simulink model "mbf_3beams"  (R2013b)
settings: Solver=VariableStepDiscrete  StartTime=0.0  StopTime=1000  RelTol=1e-3
undefined symbols (not defined in the file): pfb_coeff_gen_calc
libraries: xbsIndex_r4 x21411, casper_library_misc x306, casper_library_delays x93, casper_library_multipliers x64, casper_library_pfbs x16, simulink x16, xbsReplacements_r4 x8, xps_library x1
(part 2 of 252)

== system mbf_3beams/acc_control ==
  sync : Inport
  acc_len : Inport  Port=2
  rst : Inport  Port=3
  new_acc : Inport  Port=4
  valid : Inport  Port=5
  Counter : xbsIndex_r4/Counter  cnt_type=Count Limited  cnt_to=255  operation=Up  start_count=0  cnt_by_val=1  arith_type=Unsigned  n_bits=8  bin_pt=0  load_pin=off  rst=on  en=on  explicit_period=on  period=1  dbl_ovrd=off  use_behavioral_HDL=off  implementation=Fabric  block_type=counter
  Counter1 : xbsIndex_r4/Counter  cnt_type=Free Running  cnt_to=Inf  operation=Up  start_count=0  cnt_by_val=1  arith_type=Unsigned  n_bits=32  bin_pt=0  load_pin=off  rst=on  en=on  explicit_period=on  period=1  dbl_ovrd=off  use_behavioral_HDL=off  implementation=Fabric  block_type=counter
  acc_cntrl : Subsystem  mask{chan_bits=8}
      mask prompts: chan_bits "Number of Channels (2^?)"
  acc_control : Subsystem
  acc_count : Subsystem  Tag=xps:sw_reg  AncestorBlock=xps_library/software_register  mask(swreg){io_dir=To Processor, io_delay=0, sample_period=1, names=reg, bitwidths=32, bin_pts=0, arith_types=0, sim_port=off, show_format=off}
      mask prompts: io_dir "I/O direction"; io_delay "I/O delay"; sample_period "Sample period"; names "Bitfield names [msb...lsb]"; bitwidths "Bitfield widths"; bin_pts "Bitfield binary pts"; arith_types "Bitfield types, ufix=0, fix=1, bool=2"; sim_port "Provide sim input/output?"; show_format "Print format string?"
      mask help: A 32-bit software-accessible register. Can be divided into bitfields of varying widths and types using the fields in the block mask.
      mask init:
      | try
      |     swreg_init(gcb)
      | catch ex
      |     disp(sprintf('%s: %s', gcb, ex.message))
      |     errordlg(ex.message)
      | end
      settings: AttributesFormatString=1output
      mask TypeOptions io_dir: To Processor | From Processor
      mask Callback io_dir:
      | mask_enables = get_param(gcb, 'MaskEnables');
      | mask_names = get_param(gcb, 'MaskNames');
      | if strcmp(get_param(gcb, 'io_dir'), 'To Processor'),
      |     mask_enables{ismember(mask_names, 'sample_period')} = 'off';
      | else
      |     mask_enables{ismember(mask_names, 'sample_period')} = 'on';
      | end
      | set_param(gcb, 'MaskEnables', mask_enables);
  edge_detect : Subsystem  AncestorBlock=casper_library_misc/edge_detect  mask(edge_detect){edge=Falling, polarity=Active High, x_in=[0 0.08 0.08 0.16 0.16 0.24 0.24 0.32 0.32 0.4], y_in=[-1 -1  1  1  1  1  1  1  1  1], x_out=[0.6 0.68 0.68 0.76 0.76 0.84 0.84 0.92 0.92 1], y_out=[-1 -1  1  1 -1 -1 -1 -1 -1 -1]}
      mask prompts: edge "Edge Type"; polarity "Output Polarity"; x_in "Input X Positions"; y_in "Input Y Positions"; x_out "Output X Positions"; y_out "Output Y Positions"
      mask help: Output is active for one-cycle after each edge of the specified type (i.e. rising, falling or both).
      mask init:
      | edge_detect_init(gcb,...
      |     'edge',edge,...
      |     'polarity',polarity,...
      |     'x_in',x_in,...
      |     'x_out',x_out,...
      |     'y_in',y_in,...
      |     'y_out',y_out);
      | 
      | x_in_num=str2num(getfield(getfield(get_param(gcb,'UserData'),'parameters'),'x_in'));
      | x_out_num=str2num(getfield(getfield(get_param(gcb,'UserData'),'parameters'),'x_out'));
      | y_in_num=str2num(getfield(getfield(get_param(gcb,'UserData'),'parameters'),'y_in'));
      | y_out_num=str2num(getfield(getfield(get_param(gcb,'UserData'),'parameters'),'y_out'));
      settings: AttributesFormatString=|
      | falling edges
      | active high
      mask Help: Output is active for one-cycle after each edge of the specified type (i.e. rising, falling, or both).  Latency is zero.
      mask TypeOptions edge: Rising | Falling | Both
      mask TypeOptions polarity: Active High | Active Low
      mask Display:
      | plot(x_in_num,0.5+y_in_num*0.2);
      | plot(x_out_num,0.5+y_out_num*0.2);
  edge_detect1 : Subsystem  AncestorBlock=casper_library_misc/edge_detect  mask(edge_detect){edge=Rising, polarity=Active High, x_in=[0 0.08 0.08 0.16 0.16 0.24 0.24 0.32 0.32 0.4], y_in=[-1 -1  1  1  1  1  1  1  1  1], x_out=[0.6 0.68 0.68 0.76 0.76 0.84 0.84 0.92 0.92 1], y_out=[-1 -1  1  1 -1 -1 -1 -1 -1 -1]}
      mask prompts: edge "Edge Type"; polarity "Output Polarity"; x_in "Input X Positions"; y_in "Input Y Positions"; x_out "Output X Positions"; y_out "Output Y Positions"
      mask help: Output is active for one-cycle after each edge of the specified type (i.e. rising, falling or both).
      mask init: same as mbf_3beams/acc_control/edge_detect
      settings: AttributesFormatString=|
      | rising edges
      | active high
      mask Help: Output is active for one-cycle after each edge of the specified type (i.e. rising, falling, or both).  Latency is zero.
      mask TypeOptions edge: Rising | Falling | Both
      mask TypeOptions polarity: Active High | Active Low
      mask Display:
      | plot(x_in_num,0.5+y_in_num*0.2);
      | plot(x_out_num,0.5+y_out_num*0.2);
  edge_detect3 : Subsystem  AncestorBlock=casper_library_misc/edge_detect  mask(edge_detect){edge=Rising, polarity=Active High, x_in=[0 0.08 0.08 0.16 0.16 0.24 0.24 0.32 0.32 0.4], y_in=[-1 -1  1  1  1  1  1  1  1  1], x_out=[0.6 0.68 0.68 0.76 0.76 0.84 0.84 0.92 0.92 1], y_out=[-1 -1  1  1 -1 -1 -1 -1 -1 -1]}
      mask prompts: edge "Edge Type"; polarity "Output Polarity"; x_in "Input X Positions"; y_in "Input Y Positions"; x_out "Output X Positions"; y_out "Output Y Positions"
      mask help: Output is active for one-cycle after each edge of the specified type (i.e. rising, falling or both).
      mask init: same as mbf_3beams/acc_control/edge_detect
      settings: AttributesFormatString=|
      | rising edges
      | active high
      mask Help: Output is active for one-cycle after each edge of the specified type (i.e. rising, falling, or both).  Latency is zero.
      mask TypeOptions edge: Rising | Falling | Both
      mask TypeOptions polarity: Active High | Active Low
      mask Display:
      | plot(x_in_num,0.5+y_in_num*0.2);
      | plot(x_out_num,0.5+y_out_num*0.2);
  pipeline214 : Subsystem  AncestorBlock=casper_library_delays/pipeline  mask(pipeline){latency=1}
      mask help:
      | An explicitly laid-out delay line to avoid mapping to SRL16s
      | for use in pipelining to help achieve timing closure.
      mask init:
      | pipeline_init(gcb,'latency',latency);
      settings: AttributesFormatString=Latency = %<latency>
      mask Display:
      | port_label('input', 1, 'd');
      | port_label('output', 1, 'q');
      | disp(['Z^-', num2str(latency)]);
  pipeline50 : Subsystem  AncestorBlock=casper_library_delays/pipeline  mask(pipeline){latency=1}
      mask help:
      | An explicitly laid-out delay line to avoid mapping to SRL16s
      | for use in pipelining to help achieve timing closure.
      mask init:
      | pipeline_init(gcb,'latency',latency);
      settings: AttributesFormatString=Latency = %<latency>
      mask Display:
      | port_label('input', 1, 'd');
      | port_label('output', 1, 'q');
      | disp(['Z^-', num2str(latency)]);
  rst (dup) : InportShadow  Port=3
  ctrl_new_acc : Outport
  ctrl_addr : Outport  Port=2
  ctrl_we : Outport  Port=3
  wires:
  Counter1/1 -> acc_count/1(out_reg)
  sync/1 -> acc_cntrl/2(sync)
  acc_len/1 -> acc_cntrl/1(acc_len)
  rst/1 -> acc_cntrl/3(rst)
  acc_cntrl/1(new_acc) -> ctrl_new_acc/1
  edge_detect/1(out) -> Counter1/2
  Counter/1 -> ctrl_addr/1
  edge_detect1/1(out) -> Counter/1
  pipeline50/1(q) -> Counter/2
  pipeline214/1(q) -> ctrl_we/1
  acc_control/1(cal_we) -> edge_detect/1(in), edge_detect1/1(in), pipeline50/1(d), pipeline214/1(d)
  new_acc/1 -> edge_detect3/1(in)
  edge_detect3/1(out) -> acc_control/1(new_acc)
  valid/1 -> acc_control/2(valid)
  rst (dup)/1 -> Counter1/1

== system mbf_3beams/acc_control_bf ==
  sync : Inport
  acc_len : Inport  Port=2
  rst : Inport  Port=3
  new_acc : Inport  Port=4
  valid : Inport  Port=5
  Counter : xbsIndex_r4/Counter  cnt_type=Count Limited  cnt_to=255  operation=Up  start_count=0  cnt_by_val=1  arith_type=Unsigned  n_bits=8  bin_pt=0  load_pin=off  rst=on  en=on  explicit_period=on  period=1  dbl_ovrd=off  use_behavioral_HDL=off  implementation=Fabric  block_type=counter
  Counter1 : xbsIndex_r4/Counter  cnt_type=Free Running  cnt_to=Inf  operation=Up  start_count=0  cnt_by_val=1  arith_type=Unsigned  n_bits=32  bin_pt=0  load_pin=off  rst=on  en=on  explicit_period=on  period=1  dbl_ovrd=off  use_behavioral_HDL=off  implementation=Fabric  block_type=counter
  acc_cntrl : Subsystem  mask{chan_bits=8}
      mask prompts: chan_bits "Number of Channels (2^?)"
  acc_control : Subsystem
  acc_count : Subsystem  Tag=xps:sw_reg  AncestorBlock=xps_library/software_register  mask(swreg){io_dir=To Processor, io_delay=0, sample_period=1, names=reg, bitwidths=32, bin_pts=0, arith_types=0, sim_port=off, show_format=off}
      mask prompts: io_dir "I/O direction"; io_delay "I/O delay"; sample_period "Sample period"; names "Bitfield names [msb...lsb]"; bitwidths "Bitfield widths"; bin_pts "Bitfield binary pts"; arith_types "Bitfield types, ufix=0, fix=1, bool=2"; sim_port "Provide sim input/output?"; show_format "Print format string?"
      mask help: A 32-bit software-accessible register. Can be divided into bitfields of varying widths and types using the fields in the block mask.
      mask init:
      | try
      |     swreg_init(gcb)
      | catch ex
      |     disp(sprintf('%s: %s', gcb, ex.message))
      |     errordlg(ex.message)
      | end
      settings: AttributesFormatString=1output
      mask TypeOptions io_dir: To Processor | From Processor
      mask Callback io_dir:
      | mask_enables = get_param(gcb, 'MaskEnables');
      | mask_names = get_param(gcb, 'MaskNames');
      | if strcmp(get_param(gcb, 'io_dir'), 'To Processor'),
      |     mask_enables{ismember(mask_names, 'sample_period')} = 'off';
      | else
      |     mask_enables{ismember(mask_names, 'sample_period')} = 'on';
      | end
      | set_param(gcb, 'MaskEnables', mask_enables);
  edge_detect : Subsystem  AncestorBlock=casper_library_misc/edge_detect  mask(edge_detect){edge=Falling, polarity=Active High, x_in=[0 0.08 0.08 0.16 0.16 0.24 0.24 0.32 0.32 0.4], y_in=[-1 -1  1  1  1  1  1  1  1  1], x_out=[0.6 0.68 0.68 0.76 0.76 0.84 0.84 0.92 0.92 1], y_out=[-1 -1  1  1 -1 -1 -1 -1 -1 -1]}
      mask prompts: edge "Edge Type"; polarity "Output Polarity"; x_in "Input X Positions"; y_in "Input Y Positions"; x_out "Output X Positions"; y_out "Output Y Positions"
      mask help: Output is active for one-cycle after each edge of the specified type (i.e. rising, falling or both).
      mask init: same as mbf_3beams/acc_control/edge_detect
      settings: AttributesFormatString=|
      | falling edges
      | active high
      mask Help: Output is active for one-cycle after each edge of the specified type (i.e. rising, falling, or both).  Latency is zero.
      mask TypeOptions edge: Rising | Falling | Both
      mask TypeOptions polarity: Active High | Active Low
      mask Display:
      | plot(x_in_num,0.5+y_in_num*0.2);
      | plot(x_out_num,0.5+y_out_num*0.2);
  edge_detect1 : Subsystem  AncestorBlock=casper_library_misc/edge_detect  mask(edge_detect){edge=Rising, polarity=Active High, x_in=[0 0.08 0.08 0.16 0.16 0.24 0.24 0.32 0.32 0.4], y_in=[-1 -1  1  1  1  1  1  1  1  1], x_out=[0.6 0.68 0.68 0.76 0.76 0.84 0.84 0.92 0.92 1], y_out=[-1 -1  1  1 -1 -1 -1 -1 -1 -1]}
      mask prompts: edge "Edge Type"; polarity "Output Polarity"; x_in "Input X Positions"; y_in "Input Y Positions"; x_out "Output X Positions"; y_out "Output Y Positions"
      mask help: Output is active for one-cycle after each edge of the specified type (i.e. rising, falling or both).
      mask init: same as mbf_3beams/acc_control/edge_detect
      settings: AttributesFormatString=|
      | rising edges
      | active high
      mask Help: Output is active for one-cycle after each edge of the specified type (i.e. rising, falling, or both).  Latency is zero.
      mask TypeOptions edge: Rising | Falling | Both
      mask TypeOptions polarity: Active High | Active Low
      mask Display:
      | plot(x_in_num,0.5+y_in_num*0.2);
      | plot(x_out_num,0.5+y_out_num*0.2);
  edge_detect3 : Subsystem  AncestorBlock=casper_library_misc/edge_detect  mask(edge_detect){edge=Rising, polarity=Active High, x_in=[0 0.08 0.08 0.16 0.16 0.24 0.24 0.32 0.32 0.4], y_in=[-1 -1  1  1  1  1  1  1  1  1], x_out=[0.6 0.68 0.68 0.76 0.76 0.84 0.84 0.92 0.92 1], y_out=[-1 -1  1  1 -1 -1 -1 -1 -1 -1]}
      mask prompts: edge "Edge Type"; polarity "Output Polarity"; x_in "Input X Positions"; y_in "Input Y Positions"; x_out "Output X Positions"; y_out "Output Y Positions"
      mask help: Output is active for one-cycle after each edge of the specified type (i.e. rising, falling or both).
      mask init: same as mbf_3beams/acc_control/edge_detect
      settings: AttributesFormatString=|
      | rising edges
      | active high
      mask Help: Output is active for one-cycle after each edge of the specified type (i.e. rising, falling, or both).  Latency is zero.
      mask TypeOptions edge: Rising | Falling | Both
      mask TypeOptions polarity: Active High | Active Low
      mask Display:
      | plot(x_in_num,0.5+y_in_num*0.2);
      | plot(x_out_num,0.5+y_out_num*0.2);
  pipeline214 : Subsystem  AncestorBlock=casper_library_delays/pipeline  mask(pipeline){latency=1}
      mask help:
      | An explicitly laid-out delay line to avoid mapping to SRL16s
      | for use in pipelining to help achieve timing closure.
      mask init:
      | pipeline_init(gcb,'latency',latency);
      settings: AttributesFormatString=Latency = %<latency>
      mask Display:
      | port_label('input', 1, 'd');
      | port_label('output', 1, 'q');
      | disp(['Z^-', num2str(latency)]);
  pipeline50 : Subsystem  AncestorBlock=casper_library_delays/pipeline  mask(pipeline){latency=1}
      mask help:
      | An explicitly laid-out delay line to avoid mapping to SRL16s
      | for use in pipelining to help achieve timing closure.
      mask init:
      | pipeline_init(gcb,'latency',latency);
      settings: AttributesFormatString=Latency = %<latency>
      mask Display:
      | port_label('input', 1, 'd');
      | port_label('output', 1, 'q');
      | disp(['Z^-', num2str(latency)]);
  rst (dup) : InportShadow  Port=3
  ctrl_new_acc : Outport
  ctrl_addr : Outport  Port=2
  ctrl_we : Outport  Port=3
  wires:
  Counter1/1 -> acc_count/1(out_reg)
  sync/1 -> acc_cntrl/2(sync)
  acc_len/1 -> acc_cntrl/1(acc_len)
  rst/1 -> acc_cntrl/3(rst)
  acc_cntrl/1(new_acc) -> ctrl_new_acc/1
  edge_detect/1(out) -> Counter1/2
  Counter/1 -> ctrl_addr/1
  edge_detect1/1(out) -> Counter/1
  pipeline50/1(q) -> Counter/2
  pipeline214/1(q) -> ctrl_we/1
  acc_control/1(cal_we) -> edge_detect/1(in), edge_detect1/1(in), pipeline50/1(d), pipeline214/1(d)
  new_acc/1 -> edge_detect3/1(in)
  edge_detect3/1(out) -> acc_control/1(new_acc)
  valid/1 -> acc_control/2(valid)
  rst (dup)/1 -> Counter1/1

== system mbf_3beams/adc16x250-8 ==
  a1_sim : Inport
  a2_sim : Inport  Port=2
  a3_sim : Inport  Port=3
  a4_sim : Inport  Port=4
  b1_sim : Inport  Port=5
  b2_sim : Inport  Port=6
  b3_sim : Inport  Port=7
  b4_sim : Inport  Port=8
  c1_sim : Inport  Port=9
  c2_sim : Inport  Port=10
  c3_sim : Inport  Port=11
  c4_sim : Inport  Port=12
  d1_sim : Inport  Port=13
  d2_sim : Inport  Port=14
  d3_sim : Inport  Port=15
  d4_sim : Inport  Port=16
  mbf_3beams_adc16x250_8_a{1..4} (4 alike) : xbsIndex_r4/Gateway In  gui_display_data_type=Fixed-point  arith_type="Signed  (2's comp)"  n_bits=8  bin_pt=7  preci_type=Single  exp_width=8  frac_width=24  quantization="Round  (unbiased: +/- Inf)"  overflow=Saturate  period=1  dbl_ovrd=off  timing_constraint=None  locs_specified=off  LOCs={}  UseAsADC=off  ADCChannel='1'  inherit_from_input=off  hdl_port=on  block_type=gatewayin
  mbf_3beams_adc16x250_8_b{1..4} (4 alike) : xbsIndex_r4/Gateway In  gui_display_data_type=Fixed-point  arith_type="Signed  (2's comp)"  n_bits=8  bin_pt=7  preci_type=Single  exp_width=8  frac_width=24  quantization="Round  (unbiased: +/- Inf)"  overflow=Saturate  period=1  dbl_ovrd=off  timing_constraint=None  locs_specified=off  LOCs={}  UseAsADC=off  ADCChannel='1'  inherit_from_input=off  hdl_port=on  block_type=gatewayin
  mbf_3beams_adc16x250_8_c{1..4} (4 alike) : xbsIndex_r4/Gateway In  gui_display_data_type=Fixed-point  arith_type="Signed  (2's comp)"  n_bits=8  bin_pt=7  preci_type=Single  exp_width=8  frac_width=24  quantization="Round  (unbiased: +/- Inf)"  overflow=Saturate  period=1  dbl_ovrd=off  timing_constraint=None  locs_specified=off  LOCs={}  UseAsADC=off  ADCChannel='1'  inherit_from_input=off  hdl_port=on  block_type=gatewayin
  mbf_3beams_adc16x250_8_d{1..4} (4 alike) : xbsIndex_r4/Gateway In  gui_display_data_type=Fixed-point  arith_type="Signed  (2's comp)"  n_bits=8  bin_pt=7  preci_type=Single  exp_width=8  frac_width=24  quantization="Round  (unbiased: +/- Inf)"  overflow=Saturate  period=1  dbl_ovrd=off  timing_constraint=None  locs_specified=off  LOCs={}  UseAsADC=off  ADCChannel='1'  inherit_from_input=off  hdl_port=on  block_type=gatewayin
  a1 : Outport
  a2 : Outport  Port=2
  a3 : Outport  Port=3
  a4 : Outport  Port=4
  b1 : Outport  Port=5
  b2 : Outport  Port=6
  b3 : Outport  Port=7
  b4 : Outport  Port=8
  c1 : Outport  Port=9
  c2 : Outport  Port=10
  c3 : Outport  Port=11
  c4 : Outport  Port=12
  d1 : Outport  Port=13
  d2 : Outport  Port=14
  d3 : Outport  Port=15
  d4 : Outport  Port=16
  wires:
  a1_sim/1 -> mbf_3beams_adc16x250_8_a1/1
  mbf_3beams_adc16x250_8_a1/1 -> a1/1  "a1"
  a2_sim/1 -> mbf_3beams_adc16x250_8_a2/1
  mbf_3beams_adc16x250_8_a2/1 -> a2/1  "a2"
  a3_sim/1 -> mbf_3beams_adc16x250_8_a3/1
  mbf_3beams_adc16x250_8_a3/1 -> a3/1  "a3"
  a4_sim/1 -> mbf_3beams_adc16x250_8_a4/1
  mbf_3beams_adc16x250_8_a4/1 -> a4/1  "a4"
  b1_sim/1 -> mbf_3beams_adc16x250_8_b1/1
  mbf_3beams_adc16x250_8_b1/1 -> b1/1  "b1"
  b2_sim/1 -> mbf_3beams_adc16x250_8_b2/1
  mbf_3beams_adc16x250_8_b2/1 -> b2/1  "b2"
  b3_sim/1 -> mbf_3beams_adc16x250_8_b3/1
  mbf_3beams_adc16x250_8_b3/1 -> b3/1  "b3"
  b4_sim/1 -> mbf_3beams_adc16x250_8_b4/1
  mbf_3beams_adc16x250_8_b4/1 -> b4/1  "b4"
  c1_sim/1 -> mbf_3beams_adc16x250_8_c1/1
  mbf_3beams_adc16x250_8_c1/1 -> c1/1  "c1"
  c2_sim/1 -> mbf_3beams_adc16x250_8_c2/1
  mbf_3beams_adc16x250_8_c2/1 -> c2/1  "c2"
  c3_sim/1 -> mbf_3beams_adc16x250_8_c3/1
  mbf_3beams_adc16x250_8_c3/1 -> c3/1  "c3"
  c4_sim/1 -> mbf_3beams_adc16x250_8_c4/1
  mbf_3beams_adc16x250_8_c4/1 -> c4/1  "c4"
  d1_sim/1 -> mbf_3beams_adc16x250_8_d1/1
  mbf_3beams_adc16x250_8_d1/1 -> d1/1  "d1"
  d2_sim/1 -> mbf_3beams_adc16x250_8_d2/1
  mbf_3beams_adc16x250_8_d2/1 -> d2/1  "d2"
  d3_sim/1 -> mbf_3beams_adc16x250_8_d3/1
  mbf_3beams_adc16x250_8_d3/1 -> d3/1  "d3"
  d4_sim/1 -> mbf_3beams_adc16x250_8_d4/1
  mbf_3beams_adc16x250_8_d4/1 -> d4/1  "d4"

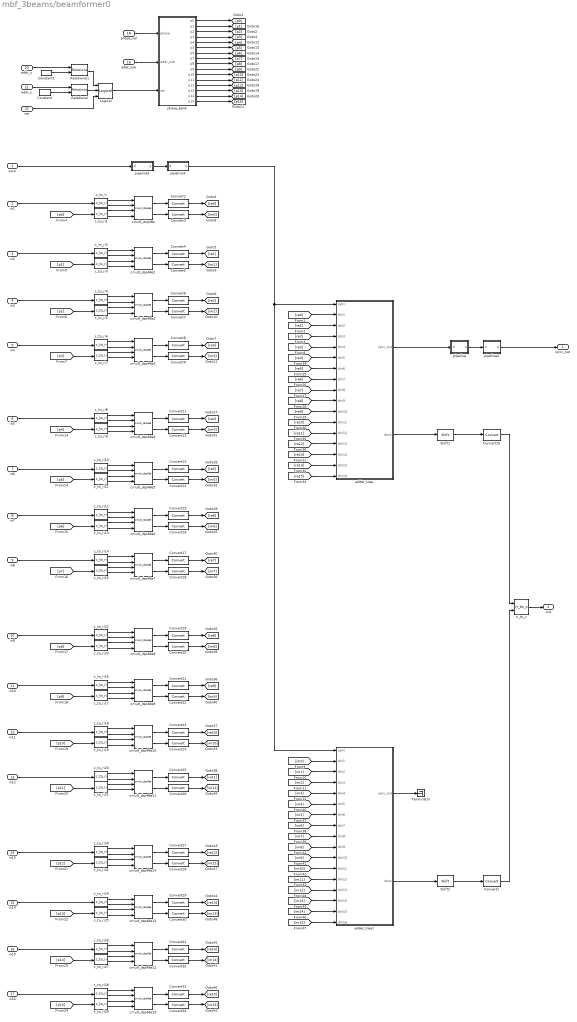
== system mbf_3beams/beamformer0 ==
  sync : Inport
  in1 : Inport  Port=2
  in2 : Inport  Port=3
  in3 : Inport  Port=4
  in4 : Inport  Port=5
  in5 : Inport  Port=6
  in6 : Inport  Port=7
  in7 : Inport  Port=8
  in8 : Inport  Port=9
  in9 : Inport  Port=10
  in10 : Inport  Port=11
  in11 : Inport  Port=12
  in12 : Inport  Port=13
  in13 : Inport  Port=14
  in14 : Inport  Port=15
  in15 : Inport  Port=16
  in16 : Inport  Port=17
  phase_val : Inport  Port=18
  addr_sub : Inport  Port=19
  addr_x : Inport  Port=20
  addr_y : Inport  Port=21
  we : Inport  Port=22
  Constant : xbsIndex_r4/Constant  const=addr_y  gui_display_data_type=Fixed-point  arith_type=Unsigned  n_bits=32  bin_pt=0  preci_type=Single  exp_width=8  frac_width=24  explicit_period=on  period=1  equ=P=C  opselect=C  inp2=PCIN>>17  opr=+  inp1=P  carry=CIN  dbl_ovrd=off  block_type=constant
  Constant1 : xbsIndex_r4/Constant  const=addr_x  gui_display_data_type=Fixed-point  arith_type=Unsigned  n_bits=32  bin_pt=0  preci_type=Single  exp_width=8  frac_width=24  explicit_period=on  period=1  equ=P=C  opselect=C  inp2=PCIN>>17  opr=+  inp1=P  carry=CIN  dbl_ovrd=off  block_type=constant
  Convert{1..34} (34 alike) : xbsIndex_r4/Convert  gui_display_data_type=Fixed-point  arith_type="Signed  (2's comp)"  n_bits=18  bin_pt=17  float_type=Single  exp_bits=8  fraction_bits=24  quantization=Truncate  overflow=Wrap  en=off  latency=2  dbl_ovrd=off  pipeline=off  block_type=convert
      settings: block_version=11.4
  Logical : xbsIndex_r4/Logical  logical_function=AND  inputs=3  en=off  latency=0  precision=Full  arith_type=Unsigned  n_bits=16  bin_pt=0  align_bp=on  dbl_ovrd=off  block_type=logical
  Relational : xbsIndex_r4/Relational  mode=a=b  en=off  latency=0  dbl_ovrd=off  block_type=relational
  Relational1 : xbsIndex_r4/Relational  mode=a=b  en=off  latency=0  dbl_ovrd=off  block_type=relational
  Shift1 : xbsIndex_r4/Shift  shift_dir=Right  shift_bits=4  en=off  latency=1  precision=Full  arith_type="Signed  (2's comp)"  n_bits=16  bin_pt=14  quantization=Truncate  overflow=Wrap  dbl_ovrd=off  block_type=shift
  Shift2 : xbsIndex_r4/Shift  shift_dir=Right  shift_bits=4  en=off  latency=1  precision=Full  arith_type="Signed  (2's comp)"  n_bits=16  bin_pt=14  quantization=Truncate  overflow=Wrap  dbl_ovrd=off  block_type=shift
  Terminator : Terminator
  adder_tree : Subsystem  AncestorBlock=casper_library_misc/adder_tree  mask(adder_tree){n_inputs=16, latency=1, adder_imp=Fabric, first_stage_hdl=on}
      mask prompts: n_inputs "Number of Inputs"; latency "Add Latency"; adder_imp "Adder implementation"; first_stage_hdl "Use behavioural HDL for first stage of adders"
      mask help:
      | Sums all inputs using a tree of adds and delays.
      |
      | Number of inputs: The number of inputs to the block to be summed
      | Add latency: The number of clock cycles to perform one addition (affects timing)..
      | Adder implementation: choice of fabric or DSP48E core, or behavioral HDL
      | Use behavioural HDL for first stage of adders: Causes the first stage of adders to be absorbed into DSP blocks if multipliers precede them (Virtex 5). Overrides Adder implementation
      mask init:
      | adder_tree_init(gcb, ...
      |     'n_inputs', n_inputs, ...
      |     'latency', latency, ...
      |     'adder_imp', adder_imp, ...
      |     'first_stage_hdl', first_stage_hdl);
      settings: AttributesFormatString=latency 4
      mask Help: eval('xlWeb(''http://casper.berkeley.edu/wiki/Adder_tree'')')
      mask TypeOptions adder_imp: Behavioral | Fabric | DSP48
  adder_tree1 : Subsystem  AncestorBlock=casper_library_misc/adder_tree  mask(adder_tree){n_inputs=16, latency=1, adder_imp=Fabric, first_stage_hdl=on}
      mask prompts: n_inputs "Number of Inputs"; latency "Add Latency"; adder_imp "Adder implementation"; first_stage_hdl "Use behavioural HDL for first stage of adders"
      mask help:
      | Sums all inputs using a tree of adds and delays.
      |
      | Number of inputs: The number of inputs to the block to be summed
      | Add latency: The number of clock cycles to perform one addition (affects timing)..
      | Adder implementation: choice of fabric or DSP48E core, or behavioral HDL
      | Use behavioural HDL for first stage of adders: Causes the first stage of adders to be absorbed into DSP blocks if multipliers precede them (Virtex 5). Overrides Adder implementation
      mask init:
      | adder_tree_init(gcb, ...
      |     'n_inputs', n_inputs, ...
      |     'latency', latency, ...
      |     'adder_imp', adder_imp, ...
      |     'first_stage_hdl', first_stage_hdl);
      settings: AttributesFormatString=latency 4
      mask Help: eval('xlWeb(''http://casper.berkeley.edu/wiki/Adder_tree'')')
      mask TypeOptions adder_imp: Behavioral | Fabric | DSP48
  c_to_ri{,1..31} (32 alike) : casper_library_misc/c_to_ri  n_bits=18  bin_pt=17
  cmult_dsp48e{,1..15} (16 alike) : casper_library_multipliers/cmult_dsp48e  n_bits_a=18  bin_pt_a=17  n_bits_b=18  bin_pt_b=17  conjugated=off  full_precision=on  n_bits_c=37  bin_pt_c=34  quantization=Truncate  overflow=Wrap  cast_latency=0
      settings: AttributesFormatString=|
      | 18_17 * 18_17 ==> 37_34
      | Latency=4
  phase_bank : Subsystem
  pipeline : Subsystem  AncestorBlock=casper_library_delays/pipeline  mask(pipeline){latency=1}
      mask help:
      | An explicitly laid-out delay line to avoid mapping to SRL16s
      | for use in pipelining to help achieve timing closure.
      mask init:
      | pipeline_init(gcb,'latency',latency);
      settings: AttributesFormatString=Latency = %<latency>
      mask Display:
      | port_label('input', 1, 'd');
      | port_label('output', 1, 'q');
      | disp(['Z^-', num2str(latency)]);
  pipeline1 : Subsystem  AncestorBlock=casper_library_delays/pipeline  mask(pipeline){latency=4}
      mask help:
      | An explicitly laid-out delay line to avoid mapping to SRL16s
      | for use in pipelining to help achieve timing closure.
      mask init:
      | pipeline_init(gcb,'latency',latency);
      settings: AttributesFormatString=Latency = %<latency>
      mask Display:
      | port_label('input', 1, 'd');
      | port_label('output', 1, 'q');
      | disp(['Z^-', num2str(latency)]);
  pipeline2 : Subsystem  AncestorBlock=casper_library_delays/pipeline  mask(pipeline){latency=2}
      mask help:
      | An explicitly laid-out delay line to avoid mapping to SRL16s
      | for use in pipelining to help achieve timing closure.
      mask init:
      | pipeline_init(gcb,'latency',latency);
      settings: AttributesFormatString=Latency = %<latency>
      mask Display:
      | port_label('input', 1, 'd');
      | port_label('output', 1, 'q');
      | disp(['Z^-', num2str(latency)]);
  pipeline3 : Subsystem  AncestorBlock=casper_library_delays/pipeline  mask(pipeline){latency=2}
      mask help:
      | An explicitly laid-out delay line to avoid mapping to SRL16s
      | for use in pipelining to help achieve timing closure.
      mask init:
      | pipeline_init(gcb,'latency',latency);
      settings: AttributesFormatString=Latency = %<latency>
      mask Display:
      | port_label('input', 1, 'd');
      | port_label('output', 1, 'q');
      | disp(['Z^-', num2str(latency)]);
  ri_to_c : casper_library_misc/ri_to_c
  sync_out : Outport
  out : Outport  Port=2
  wires:
  adder_tree1/1(sync_out) -> Terminator/1
  Constant1/1 -> Relational1/2
  Relational1/1 -> Logical/1
  addr_x/1 -> Relational1/1
  Constant/1 -> Relational/2
  Relational/1 -> Logical/2
  addr_y/1 -> Relational/1
  we/1 -> Logical/3
  [im13] -> adder_tree1/15(din14)
  [im14] -> adder_tree1/16(din15)
  [im15] -> adder_tree1/17(din16)
  [im12] -> adder_tree1/14(din13)
  [im10] -> adder_tree1/12(din11)
  [im8] -> adder_tree1/10(din9)
  [im9] -> adder_tree1/11(din10)
  [im11] -> adder_tree1/13(din12)
  [im7] -> adder_tree1/9(din8)
  [im4] -> adder_tree1/6(din5)
  [im5] -> adder_tree1/7(din6)
  [im6] -> adder_tree1/8(din7)
  [im3] -> adder_tree1/5(din4)
  [im2] -> adder_tree1/4(din3)
  [im1] -> adder_tree1/3(din2)
  [im0] -> adder_tree1/2(din1)
  pipeline3/1(q) -> adder_tree/1(sync), adder_tree1/1(sync)
  [re14] -> adder_tree/16(din15)
  [re13] -> adder_tree/15(din14)
  [re8] -> adder_tree/10(din9)
  [re5] -> adder_tree/7(din6)
  [re11] -> adder_tree/13(din12)
  [re10] -> adder_tree/12(din11)
  [re6] -> adder_tree/8(din7)
  [re4] -> adder_tree/6(din5)
  [re7] -> adder_tree/9(din8)
  [re9] -> adder_tree/11(din10)
  [re12] -> adder_tree/14(din13)
  [re15] -> adder_tree/17(din16)
  c_to_ri24/2 -> cmult_dsp48e15/2
  in14/1 -> c_to_ri24/1
  cmult_dsp48e15/1 -> Convert29/1
  Convert29/1 -> [re13]
  cmult_dsp48e15/2 -> Convert30/1
  c_to_ri24/1 -> cmult_dsp48e15/1
  c_to_ri25/2 -> cmult_dsp48e15/4
  Convert30/1 -> [im13]
  c_to_ri31/1 -> cmult_dsp48e14/3
  c_to_ri30/2 -> cmult_dsp48e14/2
  in13/1 -> c_to_ri30/1
  [p12] -> c_to_ri31/1
  cmult_dsp48e14/1 -> Convert27/1
  Convert27/1 -> [re12]
  cmult_dsp48e14/2 -> Convert28/1
  c_to_ri30/1 -> cmult_dsp48e14/1
  c_to_ri31/2 -> cmult_dsp48e14/4
  Convert28/1 -> [im12]
  c_to_ri29/1 -> cmult_dsp48e13/3
  c_to_ri28/2 -> cmult_dsp48e13/2
  in16/1 -> c_to_ri28/1
  [p15] -> c_to_ri29/1
  cmult_dsp48e13/1 -> Convert33/1
  Convert33/1 -> [re15]
  cmult_dsp48e13/2 -> Convert34/1
  c_to_ri28/1 -> cmult_dsp48e13/1
  c_to_ri29/2 -> cmult_dsp48e13/4
  Convert34/1 -> [im15]
  c_to_ri27/1 -> cmult_dsp48e12/3
  c_to_ri26/2 -> cmult_dsp48e12/2
  in15/1 -> c_to_ri26/1
  [p14] -> c_to_ri27/1
  cmult_dsp48e12/1 -> Convert31/1
  Convert31/1 -> [re14]
  cmult_dsp48e12/2 -> Convert32/1
  c_to_ri26/1 -> cmult_dsp48e12/1
  c_to_ri27/2 -> cmult_dsp48e12/4
  Convert32/1 -> [im14]
  c_to_ri25/1 -> cmult_dsp48e15/3
  [p13] -> c_to_ri25/1
  c_to_ri16/2 -> cmult_dsp48e9/2
  in10/1 -> c_to_ri16/1
  cmult_dsp48e9/1 -> Convert21/1
  Convert21/1 -> [re9]
  cmult_dsp48e9/2 -> Convert22/1
  c_to_ri16/1 -> cmult_dsp48e9/1
  c_to_ri17/2 -> cmult_dsp48e9/4
  Convert22/1 -> [im9]
  c_to_ri23/1 -> cmult_dsp48e8/3
  c_to_ri22/2 -> cmult_dsp48e8/2
  in9/1 -> c_to_ri22/1
  [p8] -> c_to_ri23/1
  cmult_dsp48e8/1 -> Convert19/1
  Convert19/1 -> [re8]
  cmult_dsp48e8/2 -> Convert20/1
  c_to_ri22/1 -> cmult_dsp48e8/1
  c_to_ri23/2 -> cmult_dsp48e8/4
  Convert20/1 -> [im8]
  c_to_ri21/1 -> cmult_dsp48e11/3
  c_to_ri20/2 -> cmult_dsp48e11/2
  in12/1 -> c_to_ri20/1
  [p11] -> c_to_ri21/1
  cmult_dsp48e11/1 -> Convert25/1
  Convert25/1 -> [re11]
  cmult_dsp48e11/2 -> Convert26/1
  c_to_ri20/1 -> cmult_dsp48e11/1
  c_to_ri21/2 -> cmult_dsp48e11/4
  Convert26/1 -> [im11]
  c_to_ri19/1 -> cmult_dsp48e10/3
  c_to_ri18/2 -> cmult_dsp48e10/2
  in11/1 -> c_to_ri18/1
  [p10] -> c_to_ri19/1
  cmult_dsp48e10/1 -> Convert23/1
  Convert23/1 -> [re10]
  cmult_dsp48e10/2 -> Convert24/1
  c_to_ri18/1 -> cmult_dsp48e10/1
  c_to_ri19/2 -> cmult_dsp48e10/4
  Convert24/1 -> [im10]
  c_to_ri17/1 -> cmult_dsp48e9/3
  [p9] -> c_to_ri17/1
  c_to_ri10/2 -> cmult_dsp48e5/2
  in6/1 -> c_to_ri10/1
  cmult_dsp48e5/1 -> Convert13/1
  Convert13/1 -> [re5]
  cmult_dsp48e5/2 -> Convert14/1
  c_to_ri10/1 -> cmult_dsp48e5/1
  c_to_ri11/2 -> cmult_dsp48e5/4
  Convert14/1 -> [im5]
  c_to_ri9/1 -> cmult_dsp48e4/3
  c_to_ri8/2 -> cmult_dsp48e4/2
  in5/1 -> c_to_ri8/1
  [p4] -> c_to_ri9/1
  cmult_dsp48e4/1 -> Convert11/1
  Convert11/1 -> [re4]
  cmult_dsp48e4/2 -> Convert12/1
  c_to_ri8/1 -> cmult_dsp48e4/1
  c_to_ri9/2 -> cmult_dsp48e4/4
  Convert12/1 -> [im4]
  c_to_ri15/1 -> cmult_dsp48e7/3
  c_to_ri14/2 -> cmult_dsp48e7/2
  in8/1 -> c_to_ri14/1
  [p7] -> c_to_ri15/1
  cmult_dsp48e7/1 -> Convert17/1
  Convert17/1 -> [re7]
  cmult_dsp48e7/2 -> Convert18/1
  c_to_ri14/1 -> cmult_dsp48e7/1
  c_to_ri15/2 -> cmult_dsp48e7/4
  Convert18/1 -> [im7]
  c_to_ri13/1 -> cmult_dsp48e6/3
  c_to_ri12/2 -> cmult_dsp48e6/2
  in7/1 -> c_to_ri12/1
  [p6] -> c_to_ri13/1
  cmult_dsp48e6/1 -> Convert15/1
  Convert15/1 -> [re6]
  cmult_dsp48e6/2 -> Convert16/1
  c_to_ri12/1 -> cmult_dsp48e6/1
  c_to_ri13/2 -> cmult_dsp48e6/4
  Convert16/1 -> [im6]
  c_to_ri11/1 -> cmult_dsp48e5/3
  [p5] -> c_to_ri11/1
  Logical/1 -> phase_bank/3(we)
  addr_sub/1 -> phase_bank/2(addr_sub)
  phase_val/1 -> phase_bank/1(phase)
  ri_to_c/1 -> out/1
  pipeline2/1(q) -> sync_out/1
  Convert10/1 -> ri_to_c/1
  Convert1/1 -> ri_to_c/2
  Shift2/1 -> Convert1/1
  Shift1/1 -> Convert10/1
  pipeline/1(q) -> pipeline2/1(d)
  [re0] -> adder_tree/2(din1)
  [re1] -> adder_tree/3(din2)
  [re2] -> adder_tree/4(din3)
  [re3] -> adder_tree/5(din4)
  adder_tree/1(sync_out) -> pipeline/1(d)
  adder_tree1/2(dout) -> Shift2/1
  adder_tree/2(dout) -> Shift1/1
  c_to_ri2/2 -> cmult_dsp48e1/2
  in2/1 -> c_to_ri2/1
  cmult_dsp48e1/1 -> Convert4/1
  Convert4/1 -> [re1]
  cmult_dsp48e1/2 -> Convert5/1
  c_to_ri2/1 -> cmult_dsp48e1/1
  c_to_ri3/2 -> cmult_dsp48e1/4
  Convert5/1 -> [im1]
  c_to_ri1/1 -> cmult_dsp48e/3
  c_to_ri/2 -> cmult_dsp48e/2
  in1/1 -> c_to_ri/1
  [p0] -> c_to_ri1/1
  cmult_dsp48e/1 -> Convert2/1
  Convert2/1 -> [re0]
  cmult_dsp48e/2 -> Convert3/1
  c_to_ri/1 -> cmult_dsp48e/1
  c_to_ri1/2 -> cmult_dsp48e/4
  Convert3/1 -> [im0]
  pipeline1/1(q) -> pipeline3/1(d)
  sync/1 -> pipeline1/1(d)
  c_to_ri7/1 -> cmult_dsp48e3/3
  c_to_ri6/2 -> cmult_dsp48e3/2
  in4/1 -> c_to_ri6/1
  [p3] -> c_to_ri7/1
  cmult_dsp48e3/1 -> Convert8/1
  Convert8/1 -> [re3]
  cmult_dsp48e3/2 -> Convert9/1
  c_to_ri6/1 -> cmult_dsp48e3/1
  c_to_ri7/2 -> cmult_dsp48e3/4
  Convert9/1 -> [im3]
  c_to_ri5/1 -> cmult_dsp48e2/3
  c_to_ri4/2 -> cmult_dsp48e2/2
  in3/1 -> c_to_ri4/1
  [p2] -> c_to_ri5/1
  cmult_dsp48e2/1 -> Convert6/1
  Convert6/1 -> [re2]
  cmult_dsp48e2/2 -> Convert7/1
  c_to_ri4/1 -> cmult_dsp48e2/1
  c_to_ri5/2 -> cmult_dsp48e2/4
  Convert7/1 -> [im2]
  c_to_ri3/1 -> cmult_dsp48e1/3
  [p1] -> c_to_ri3/1
  phase_bank/1(p0) -> [p0]
  phase_bank/2(p1) -> [p1]
  phase_bank/3(p2) -> [p2]
  phase_bank/4(p3) -> [p3]
  phase_bank/5(p4) -> [p4]
  phase_bank/6(p5) -> [p5]
  phase_bank/7(p6) -> [p6]
  phase_bank/8(p7) -> [p7]
  phase_bank/9(p8) -> [p8]
  phase_bank/10(p9) -> [p9]
  phase_bank/11(p10) -> [p10]
  phase_bank/12(p11) -> [p11]
  phase_bank/13(p12) -> [p12]
  phase_bank/14(p13) -> [p13]
  phase_bank/15(p14) -> [p14]
  phase_bank/16(p15) -> [p15]

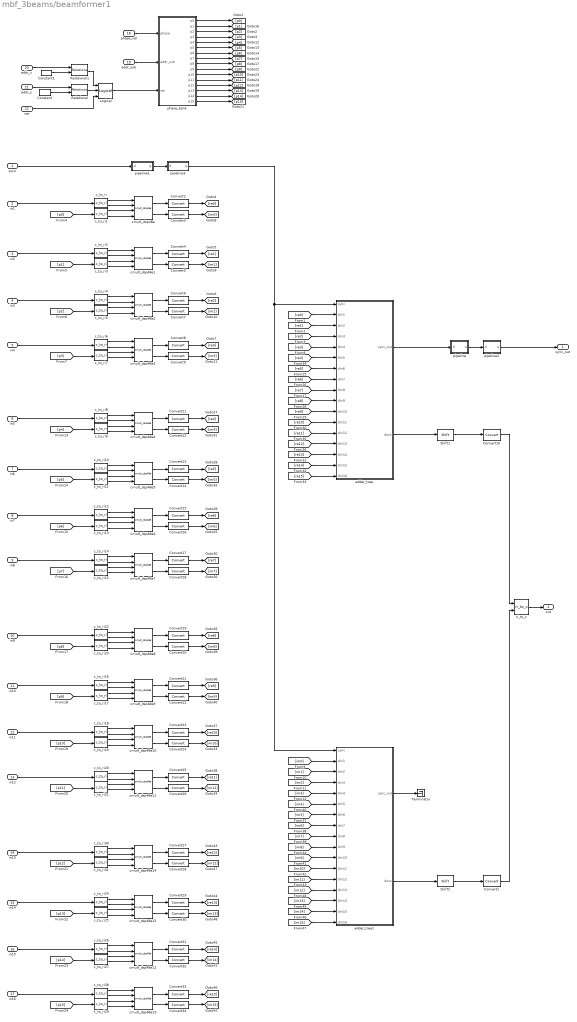
== system mbf_3beams/beamformer1 ==
  sync : Inport
  in1 : Inport  Port=2
  in2 : Inport  Port=3
  in3 : Inport  Port=4
  in4 : Inport  Port=5
  in5 : Inport  Port=6
  in6 : Inport  Port=7
  in7 : Inport  Port=8
  in8 : Inport  Port=9
  in9 : Inport  Port=10
  in10 : Inport  Port=11
  in11 : Inport  Port=12
  in12 : Inport  Port=13
  in13 : Inport  Port=14
  in14 : Inport  Port=15
  in15 : Inport  Port=16
  in16 : Inport  Port=17
  phase_val : Inport  Port=18
  addr_sub : Inport  Port=19
  addr_x : Inport  Port=20
  addr_y : Inport  Port=21
  we : Inport  Port=22
  Constant : xbsIndex_r4/Constant  const=addr_y  gui_display_data_type=Fixed-point  arith_type=Unsigned  n_bits=32  bin_pt=0  preci_type=Single  exp_width=8  frac_width=24  explicit_period=on  period=1  equ=P=C  opselect=C  inp2=PCIN>>17  opr=+  inp1=P  carry=CIN  dbl_ovrd=off  block_type=constant
  Constant1 : xbsIndex_r4/Constant  const=addr_x  gui_display_data_type=Fixed-point  arith_type=Unsigned  n_bits=32  bin_pt=0  preci_type=Single  exp_width=8  frac_width=24  explicit_period=on  period=1  equ=P=C  opselect=C  inp2=PCIN>>17  opr=+  inp1=P  carry=CIN  dbl_ovrd=off  block_type=constant
  Convert{1..34} (34 alike) : xbsIndex_r4/Convert  gui_display_data_type=Fixed-point  arith_type="Signed  (2's comp)"  n_bits=18  bin_pt=17  float_type=Single  exp_bits=8  fraction_bits=24  quantization=Truncate  overflow=Wrap  en=off  latency=2  dbl_ovrd=off  pipeline=off  block_type=convert
      settings: block_version=11.4
  Logical : xbsIndex_r4/Logical  logical_function=AND  inputs=3  en=off  latency=0  precision=Full  arith_type=Unsigned  n_bits=16  bin_pt=0  align_bp=on  dbl_ovrd=off  block_type=logical
  Relational : xbsIndex_r4/Relational  mode=a=b  en=off  latency=0  dbl_ovrd=off  block_type=relational
  Relational1 : xbsIndex_r4/Relational  mode=a=b  en=off  latency=0  dbl_ovrd=off  block_type=relational
  Shift1 : xbsIndex_r4/Shift  shift_dir=Right  shift_bits=4  en=off  latency=1  precision=Full  arith_type="Signed  (2's comp)"  n_bits=16  bin_pt=14  quantization=Truncate  overflow=Wrap  dbl_ovrd=off  block_type=shift
  Shift2 : xbsIndex_r4/Shift  shift_dir=Right  shift_bits=4  en=off  latency=1  precision=Full  arith_type="Signed  (2's comp)"  n_bits=16  bin_pt=14  quantization=Truncate  overflow=Wrap  dbl_ovrd=off  block_type=shift
  Terminator : Terminator
  adder_tree : Subsystem  AncestorBlock=casper_library_misc/adder_tree  mask(adder_tree){n_inputs=16, latency=1, adder_imp=Fabric, first_stage_hdl=on}
      mask prompts: n_inputs "Number of Inputs"; latency "Add Latency"; adder_imp "Adder implementation"; first_stage_hdl "Use behavioural HDL for first stage of adders"
      mask help:
      | Sums all inputs using a tree of adds and delays.
      |
      | Number of inputs: The number of inputs to the block to be summed
      | Add latency: The number of clock cycles to perform one addition (affects timing)..
      | Adder implementation: choice of fabric or DSP48E core, or behavioral HDL
      | Use behavioural HDL for first stage of adders: Causes the first stage of adders to be absorbed into DSP blocks if multipliers precede them (Virtex 5). Overrides Adder implementation
      mask init:
      | adder_tree_init(gcb, ...
      |     'n_inputs', n_inputs, ...
      |     'latency', latency, ...
      |     'adder_imp', adder_imp, ...
      |     'first_stage_hdl', first_stage_hdl);
      settings: AttributesFormatString=latency 4
      mask Help: eval('xlWeb(''http://casper.berkeley.edu/wiki/Adder_tree'')')
      mask TypeOptions adder_imp: Behavioral | Fabric | DSP48
  adder_tree1 : Subsystem  AncestorBlock=casper_library_misc/adder_tree  mask(adder_tree){n_inputs=16, latency=1, adder_imp=Fabric, first_stage_hdl=on}
      mask prompts: n_inputs "Number of Inputs"; latency "Add Latency"; adder_imp "Adder implementation"; first_stage_hdl "Use behavioural HDL for first stage of adders"
      mask help:
      | Sums all inputs using a tree of adds and delays.
      |
      | Number of inputs: The number of inputs to the block to be summed
      | Add latency: The number of clock cycles to perform one addition (affects timing)..
      | Adder implementation: choice of fabric or DSP48E core, or behavioral HDL
      | Use behavioural HDL for first stage of adders: Causes the first stage of adders to be absorbed into DSP blocks if multipliers precede them (Virtex 5). Overrides Adder implementation
      mask init:
      | adder_tree_init(gcb, ...
      |     'n_inputs', n_inputs, ...
      |     'latency', latency, ...
      |     'adder_imp', adder_imp, ...
      |     'first_stage_hdl', first_stage_hdl);
      settings: AttributesFormatString=latency 4
      mask Help: eval('xlWeb(''http://casper.berkeley.edu/wiki/Adder_tree'')')
      mask TypeOptions adder_imp: Behavioral | Fabric | DSP48
  c_to_ri{,1..31} (32 alike) : casper_library_misc/c_to_ri  n_bits=18  bin_pt=17
  cmult_dsp48e{,1..15} (16 alike) : casper_library_multipliers/cmult_dsp48e  n_bits_a=18  bin_pt_a=17  n_bits_b=18  bin_pt_b=17  conjugated=off  full_precision=on  n_bits_c=37  bin_pt_c=34  quantization=Truncate  overflow=Wrap  cast_latency=0
      settings: AttributesFormatString=|
      | 18_17 * 18_17 ==> 37_34
      | Latency=4
  phase_bank : Subsystem
  pipeline : Subsystem  AncestorBlock=casper_library_delays/pipeline  mask(pipeline){latency=1}
      mask help:
      | An explicitly laid-out delay line to avoid mapping to SRL16s
      | for use in pipelining to help achieve timing closure.
      mask init:
      | pipeline_init(gcb,'latency',latency);
      settings: AttributesFormatString=Latency = %<latency>
      mask Display:
      | port_label('input', 1, 'd');
      | port_label('output', 1, 'q');
      | disp(['Z^-', num2str(latency)]);
  pipeline1 : Subsystem  AncestorBlock=casper_library_delays/pipeline  mask(pipeline){latency=4}
      mask help:
      | An explicitly laid-out delay line to avoid mapping to SRL16s
      | for use in pipelining to help achieve timing closure.
      mask init:
      | pipeline_init(gcb,'latency',latency);
      settings: AttributesFormatString=Latency = %<latency>
      mask Display:
      | port_label('input', 1, 'd');
      | port_label('output', 1, 'q');
      | disp(['Z^-', num2str(latency)]);
  pipeline2 : Subsystem  AncestorBlock=casper_library_delays/pipeline  mask(pipeline){latency=2}
      mask help:
      | An explicitly laid-out delay line to avoid mapping to SRL16s
      | for use in pipelining to help achieve timing closure.
      mask init:
      | pipeline_init(gcb,'latency',latency);
      settings: AttributesFormatString=Latency = %<latency>
      mask Display:
      | port_label('input', 1, 'd');
      | port_label('output', 1, 'q');
      | disp(['Z^-', num2str(latency)]);
  pipeline3 : Subsystem  AncestorBlock=casper_library_delays/pipeline  mask(pipeline){latency=2}
      mask help:
      | An explicitly laid-out delay line to avoid mapping to SRL16s
      | for use in pipelining to help achieve timing closure.
      mask init:
      | pipeline_init(gcb,'latency',latency);
      settings: AttributesFormatString=Latency = %<latency>
      mask Display:
      | port_label('input', 1, 'd');
      | port_label('output', 1, 'q');
      | disp(['Z^-', num2str(latency)]);
  ri_to_c : casper_library_misc/ri_to_c
  sync_out : Outport
  out : Outport  Port=2
  wires:
  adder_tree1/1(sync_out) -> Terminator/1
  Constant1/1 -> Relational1/2
  Relational1/1 -> Logical/1
  addr_x/1 -> Relational1/1
  Constant/1 -> Relational/2
  Relational/1 -> Logical/2
  addr_y/1 -> Relational/1
  we/1 -> Logical/3
  [im13] -> adder_tree1/15(din14)
  [im14] -> adder_tree1/16(din15)
  [im15] -> adder_tree1/17(din16)
  [im12] -> adder_tree1/14(din13)
  [im10] -> adder_tree1/12(din11)
  [im8] -> adder_tree1/10(din9)
  [im9] -> adder_tree1/11(din10)
  [im11] -> adder_tree1/13(din12)
  [im7] -> adder_tree1/9(din8)
  [im4] -> adder_tree1/6(din5)
  [im5] -> adder_tree1/7(din6)
  [im6] -> adder_tree1/8(din7)
  [im3] -> adder_tree1/5(din4)
  [im2] -> adder_tree1/4(din3)
  [im1] -> adder_tree1/3(din2)
  [im0] -> adder_tree1/2(din1)
  pipeline3/1(q) -> adder_tree/1(sync), adder_tree1/1(sync)
  [re14] -> adder_tree/16(din15)
  [re13] -> adder_tree/15(din14)
  [re8] -> adder_tree/10(din9)
  [re5] -> adder_tree/7(din6)
  [re11] -> adder_tree/13(din12)
  [re10] -> adder_tree/12(din11)
  [re6] -> adder_tree/8(din7)
  [re4] -> adder_tree/6(din5)
  [re7] -> adder_tree/9(din8)
  [re9] -> adder_tree/11(din10)
  [re12] -> adder_tree/14(din13)
  [re15] -> adder_tree/17(din16)
  c_to_ri24/2 -> cmult_dsp48e15/2
  in14/1 -> c_to_ri24/1
  cmult_dsp48e15/1 -> Convert29/1
  Convert29/1 -> [re13]
  cmult_dsp48e15/2 -> Convert30/1
  c_to_ri24/1 -> cmult_dsp48e15/1
  c_to_ri25/2 -> cmult_dsp48e15/4
  Convert30/1 -> [im13]
  c_to_ri31/1 -> cmult_dsp48e14/3
  c_to_ri30/2 -> cmult_dsp48e14/2
  in13/1 -> c_to_ri30/1
  [p12] -> c_to_ri31/1
  cmult_dsp48e14/1 -> Convert27/1
  Convert27/1 -> [re12]
  cmult_dsp48e14/2 -> Convert28/1
  c_to_ri30/1 -> cmult_dsp48e14/1
  c_to_ri31/2 -> cmult_dsp48e14/4
  Convert28/1 -> [im12]
  c_to_ri29/1 -> cmult_dsp48e13/3
  c_to_ri28/2 -> cmult_dsp48e13/2
  in16/1 -> c_to_ri28/1
  [p15] -> c_to_ri29/1
  cmult_dsp48e13/1 -> Convert33/1
  Convert33/1 -> [re15]
  cmult_dsp48e13/2 -> Convert34/1
  c_to_ri28/1 -> cmult_dsp48e13/1
  c_to_ri29/2 -> cmult_dsp48e13/4
  Convert34/1 -> [im15]
  c_to_ri27/1 -> cmult_dsp48e12/3
  c_to_ri26/2 -> cmult_dsp48e12/2
  in15/1 -> c_to_ri26/1
  [p14] -> c_to_ri27/1
  cmult_dsp48e12/1 -> Convert31/1
  Convert31/1 -> [re14]
  cmult_dsp48e12/2 -> Convert32/1
  c_to_ri26/1 -> cmult_dsp48e12/1
  c_to_ri27/2 -> cmult_dsp48e12/4
  Convert32/1 -> [im14]
  c_to_ri25/1 -> cmult_dsp48e15/3
  [p13] -> c_to_ri25/1
  c_to_ri16/2 -> cmult_dsp48e9/2
  in10/1 -> c_to_ri16/1
  cmult_dsp48e9/1 -> Convert21/1
  Convert21/1 -> [re9]
  cmult_dsp48e9/2 -> Convert22/1
  c_to_ri16/1 -> cmult_dsp48e9/1
  c_to_ri17/2 -> cmult_dsp48e9/4
  Convert22/1 -> [im9]
  c_to_ri23/1 -> cmult_dsp48e8/3
  c_to_ri22/2 -> cmult_dsp48e8/2
  in9/1 -> c_to_ri22/1
  [p8] -> c_to_ri23/1
  cmult_dsp48e8/1 -> Convert19/1
  Convert19/1 -> [re8]
  cmult_dsp48e8/2 -> Convert20/1
  c_to_ri22/1 -> cmult_dsp48e8/1
  c_to_ri23/2 -> cmult_dsp48e8/4
  Convert20/1 -> [im8]
  c_to_ri21/1 -> cmult_dsp48e11/3
  c_to_ri20/2 -> cmult_dsp48e11/2
  in12/1 -> c_to_ri20/1
  [p11] -> c_to_ri21/1
  cmult_dsp48e11/1 -> Convert25/1
  Convert25/1 -> [re11]
  cmult_dsp48e11/2 -> Convert26/1
  c_to_ri20/1 -> cmult_dsp48e11/1
  c_to_ri21/2 -> cmult_dsp48e11/4
  Convert26/1 -> [im11]
  c_to_ri19/1 -> cmult_dsp48e10/3
  c_to_ri18/2 -> cmult_dsp48e10/2
  in11/1 -> c_to_ri18/1
  [p10] -> c_to_ri19/1
  cmult_dsp48e10/1 -> Convert23/1
  Convert23/1 -> [re10]
  cmult_dsp48e10/2 -> Convert24/1
  c_to_ri18/1 -> cmult_dsp48e10/1
  c_to_ri19/2 -> cmult_dsp48e10/4
  Convert24/1 -> [im10]
  c_to_ri17/1 -> cmult_dsp48e9/3
  [p9] -> c_to_ri17/1
  c_to_ri10/2 -> cmult_dsp48e5/2
  in6/1 -> c_to_ri10/1
  cmult_dsp48e5/1 -> Convert13/1
  Convert13/1 -> [re5]
  cmult_dsp48e5/2 -> Convert14/1
  c_to_ri10/1 -> cmult_dsp48e5/1
  c_to_ri11/2 -> cmult_dsp48e5/4
  Convert14/1 -> [im5]
  c_to_ri9/1 -> cmult_dsp48e4/3
  c_to_ri8/2 -> cmult_dsp48e4/2
  in5/1 -> c_to_ri8/1
  [p4] -> c_to_ri9/1
  cmult_dsp48e4/1 -> Convert11/1
  Convert11/1 -> [re4]
  cmult_dsp48e4/2 -> Convert12/1
  c_to_ri8/1 -> cmult_dsp48e4/1
  c_to_ri9/2 -> cmult_dsp48e4/4
  Convert12/1 -> [im4]
  c_to_ri15/1 -> cmult_dsp48e7/3
  c_to_ri14/2 -> cmult_dsp48e7/2
  in8/1 -> c_to_ri14/1
  [p7] -> c_to_ri15/1
  cmult_dsp48e7/1 -> Convert17/1
  Convert17/1 -> [re7]
  cmult_dsp48e7/2 -> Convert18/1
  c_to_ri14/1 -> cmult_dsp48e7/1
  c_to_ri15/2 -> cmult_dsp48e7/4
  Convert18/1 -> [im7]
  c_to_ri13/1 -> cmult_dsp48e6/3
  c_to_ri12/2 -> cmult_dsp48e6/2
  in7/1 -> c_to_ri12/1
  [p6] -> c_to_ri13/1
  cmult_dsp48e6/1 -> Convert15/1
  Convert15/1 -> [re6]
  cmult_dsp48e6/2 -> Convert16/1
  c_to_ri12/1 -> cmult_dsp48e6/1
  c_to_ri13/2 -> cmult_dsp48e6/4
  Convert16/1 -> [im6]
  c_to_ri11/1 -> cmult_dsp48e5/3
  [p5] -> c_to_ri11/1
  Logical/1 -> phase_bank/3(we)
  addr_sub/1 -> phase_bank/2(addr_sub)
  phase_val/1 -> phase_bank/1(phase)
  ri_to_c/1 -> out/1
  pipeline2/1(q) -> sync_out/1
  Convert10/1 -> ri_to_c/1
  Convert1/1 -> ri_to_c/2
  Shift2/1 -> Convert1/1
  Shift1/1 -> Convert10/1
  pipeline/1(q) -> pipeline2/1(d)
  [re0] -> adder_tree/2(din1)
  [re1] -> adder_tree/3(din2)
  [re2] -> adder_tree/4(din3)
  [re3] -> adder_tree/5(din4)
  adder_tree/1(sync_out) -> pipeline/1(d)
  adder_tree1/2(dout) -> Shift2/1
  adder_tree/2(dout) -> Shift1/1
  c_to_ri2/2 -> cmult_dsp48e1/2
  in2/1 -> c_to_ri2/1
  cmult_dsp48e1/1 -> Convert4/1
  Convert4/1 -> [re1]
  cmult_dsp48e1/2 -> Convert5/1
  c_to_ri2/1 -> cmult_dsp48e1/1
  c_to_ri3/2 -> cmult_dsp48e1/4
  Convert5/1 -> [im1]
  c_to_ri1/1 -> cmult_dsp48e/3
  c_to_ri/2 -> cmult_dsp48e/2
  in1/1 -> c_to_ri/1
  [p0] -> c_to_ri1/1
  cmult_dsp48e/1 -> Convert2/1
  Convert2/1 -> [re0]
  cmult_dsp48e/2 -> Convert3/1
  c_to_ri/1 -> cmult_dsp48e/1
  c_to_ri1/2 -> cmult_dsp48e/4
  Convert3/1 -> [im0]
  pipeline1/1(q) -> pipeline3/1(d)
  sync/1 -> pipeline1/1(d)
  c_to_ri7/1 -> cmult_dsp48e3/3
  c_to_ri6/2 -> cmult_dsp48e3/2
  in4/1 -> c_to_ri6/1
  [p3] -> c_to_ri7/1
  cmult_dsp48e3/1 -> Convert8/1
  Convert8/1 -> [re3]
  cmult_dsp48e3/2 -> Convert9/1
  c_to_ri6/1 -> cmult_dsp48e3/1
  c_to_ri7/2 -> cmult_dsp48e3/4
  Convert9/1 -> [im3]
  c_to_ri5/1 -> cmult_dsp48e2/3
  c_to_ri4/2 -> cmult_dsp48e2/2
  in3/1 -> c_to_ri4/1
  [p2] -> c_to_ri5/1
  cmult_dsp48e2/1 -> Convert6/1
  Convert6/1 -> [re2]
  cmult_dsp48e2/2 -> Convert7/1
  c_to_ri4/1 -> cmult_dsp48e2/1
  c_to_ri5/2 -> cmult_dsp48e2/4
  Convert7/1 -> [im2]
  c_to_ri3/1 -> cmult_dsp48e1/3
  [p1] -> c_to_ri3/1
  phase_bank/1(p0) -> [p0]
  phase_bank/2(p1) -> [p1]
  phase_bank/3(p2) -> [p2]
  phase_bank/4(p3) -> [p3]
  phase_bank/5(p4) -> [p4]
  phase_bank/6(p5) -> [p5]
  phase_bank/7(p6) -> [p6]
  phase_bank/8(p7) -> [p7]
  phase_bank/9(p8) -> [p8]
  phase_bank/10(p9) -> [p9]
  phase_bank/11(p10) -> [p10]
  phase_bank/12(p11) -> [p11]
  phase_bank/13(p12) -> [p12]
  phase_bank/14(p13) -> [p13]
  phase_bank/15(p14) -> [p14]
  phase_bank/16(p15) -> [p15]
